simulink model "ofdm_txrx_mimo"  (Simulink 7.1)
settings: Solver=ode45  StartTime=0.0  StopTime=6e3  RelTol=1e-3
PreLoadFcn:
  | ofdm_rx_mimo_init
InitFcn:
  | ofdm_rx_mimo_init
StartFcn:
  | ofdm_rx_mimo_init
values from neighbouring files (symbols this model uses):
  BER_RAM_init_size = 2048    [ofdm_tx_mimo_init.m]
  CPLength = 16    [ofdm_tx_mimo_init.m]
  CRCPolynomial = hex2dec('04c11db7')    [ofdm_tx_mimo_init.m]
  freqOffset_track_filtCoef_i = hex2dec('2500')/2^32    [ofdm_rx_mimo_init.m]
  freqOffset_track_filtCoef_p = hex2dec('d0000')/2^32    [ofdm_rx_mimo_init.m]
  hi = zeros(1,length(cci))    [ofdm_rx_mimo_init.m]
  hr = zeros(1,length(ccr))    [ofdm_rx_mimo_init.m]
  max_CP_length = 16    [ofdm_tx_mimo_init.m]
  max_num_baseRateSymbols = 31    [ofdm_tx_mimo_init.m]
  max_num_subcarriers = 64    [ofdm_tx_mimo_init.m]
  max_num_trainingSymbols = 15    [ofdm_tx_mimo_init.m]
  max_numBytes = 16384    [ofdm_tx_mimo_init.m]
  max_OFDM_symbols = 2047    [ofdm_tx_mimo_init.m]
  modConstellation_qam16 = .75*[1 3 -1 -3]./3    [ofdm_tx_mimo_init.m]
  modConstellation_qam256 = 0.9375*[3 1 5 7 11 9 13 15 -3 -1 -5 -7 -11 -9 -13 -15]./15    [ofdm_tx_mimo_init.m]
  modConstellation_qam64 = 0.875*[3 1 5 7 -3 -1 -5 -7]./7    [ofdm_tx_mimo_init.m]
  modConstellation_qpsk = [1 -1]./sqrt(2)    [ofdm_tx_mimo_init.m]
  numBaseRateSymbols = 2    [ofdm_tx_mimo_init.m]
  numSubcarriers = 64    [ofdm_tx_mimo_init.m]
  numTrainingSymbols = 2    [ofdm_tx_mimo_init.m]
  pilot1_indicies = 7 + ( (64-7) * 2^16)    (= 3735559)    [ofdm_tx_mimo_init.m]
  pilot2_indicies = 21 + ( (64-21) * 2^16)    (= 2818069)    [ofdm_tx_mimo_init.m]
  pilotValue_neg = hex2dec('8000') + (2^16 * 0)    [ofdm_tx_mimo_init.m]
  pilotValue_pos = hex2dec('7FFF') + (2^16 * 0)    [ofdm_tx_mimo_init.m]
  pkt_crossCorr_thresh = 0.7    [ofdm_rx_mimo_init.m]
  pkt_energy_thresh = 0    [ofdm_rx_mimo_init.m]
  pkt_numPayloadBytes = 1400+42-6    (= 1436)    [ofdm_tx_mimo_init.m]
  pktByteNums = numHeaderBytes +  				byteIndex_numPayloadBytes(1)*2^8 +  				byteIndex_numPayloadBytes(2)*2^16 +  				byteIndex_simpleDynModMasks * 2^24    [ofdm_rx_mimo_init.m]
  PktDet_Delay = 26 + Symbol_Timing_Offset*2^7    (= 1050)    [ofdm_rx_mimo_init.m]
  preamble = 6*[0 shortsyms_10 longsyms_2]    [ofdm_tx_mimo_init.m]
  preamble_I = real(preamble)    [ofdm_tx_mimo_init.m]
  preamble_Q = imag(preamble)    [ofdm_tx_mimo_init.m]
  RAM_init_size = max_numBytes    (= 16384)    [ofdm_tx_mimo_init.m]
  reg_Interrupt_PktBuf_Control = reg_InterruptControl +  	1 * 2^16 +  	0 * 2^24    (= 65656)    [ofdm_rx_mimo_init.m]
  rx_controlBits = 1 * 2  		 + 1 * 4  		 + 0 * 8  		 + 1 * 16 * rx_SISO_Mode  		 + 1 * 32  		 + 1 * 64  		 + 0 * 128  		 + 1 * 256  		 + 0 * 512  		 + 1 * 1024  		 + 0 * 2048  		 + 1 * 4096  		 + 1 * 8192  		 + 0 * 16384  		 + 1 * 65536  		 + 1 * 131072  		 + 0 * 262144  		 + 1 * 524288    [ofdm_rx_mimo_init.m]
  Rx_PhaseNoiseTrack_K = 0.6094    [ofdm_rx_mimo_init.m]
  rx_postEq_scaling = round(rxScaling*2^11) * (1 + 2^16)    [ofdm_rx_mimo_init.m]
  RxDataScrambling_Seq = [40 198 78 63 82 173 102 245 48 111 172 115 147 230 216 93 72 65 62 2 205 242 122 90 128 83 105 97 73 10 5 252]    [ofdm_tx_mimo_init.m]
  simOnly_numSamples = length(preamble)+( (numSubcarriers+CPLength)*(numTrainingSymbols + numBaseRateSymbols + numFullRateSymbols/2) )    [ofdm_tx_mimo_init.m]
  train = [train train]    [ofdm_tx_mimo_init.m]
  tx_controlBits = (antB_preambleShift * 2^4) + (2^0 * tx_SISO_Mode) + 4*0 + 8*1    (= 73)    [ofdm_tx_mimo_init.m]
  TxDataScrambling_Seq = [40 198 78 63 82 173 102 245 48 111 172 115 147 230 216 93 72 65 62 2 205 242 122 90 128 83 105 97 73 10 5 252]    [ofdm_tx_mimo_init.m]
  txReg_symbolCounts = (2^16 * numFullRateSymbols) + (2^8 *numBaseRateSymbols ) + numTrainingSymbols    [ofdm_tx_mimo_init.m]
  TxRx_FFTScaling = bin2dec('010111') + (bin2dec('000101') * 2^6)    [ofdm_tx_mimo_init.m]
undefined symbols (not defined in the file or in its folder): AntA, AntB, BaseRate, CRC_table_gen, gcbh, intfilt, pktTiming_count_payloadStart, pktTiming_symbol_length, xlBlockLoadCallback, xlGetSimulinkPeriod
libraries: xbsIndex_r4 x4212, dspmlti4 x4, dspsigops x4, commchan3 x2, simulink x2, commrflib2 x2, dspsrcs4 x1
(part 6 of 43)

== system ofdm_txrx_mimo/OFDM Tx MIMO/OutputMuxes ==
  note: This block adds the preamble onto the signal
      | according to what the preamble generator block sends it.
  note: Cheap output-enable circuit. Output registers are
      | brought out of reset on the first 0->1 transition in the
      | LSB of Pream Index. In other words, the outputs are
      | enabled when the preamble starts, and disabled when
      | the entire packet is finished.
  note: If CP length is 16, we don't need delay here.
      | But if we have CP less than 16, cause RAMS reading address happens later,
      | so does the data. Then data and Sel are pushed further away from preamble.
  Pream Index : Inport  OutDataType=sfix(16)  OutScaling=2^0
  AntA I : Inport  Port=2  OutDataType=sfix(16)  OutScaling=2^0
  AntA Q : Inport  Port=3  OutDataType=sfix(16)  OutScaling=2^0
  AntB I : Inport  Port=4  OutDataType=sfix(16)  OutScaling=2^0
  AntB Q : Inport  Port=5  OutDataType=sfix(16)  OutScaling=2^0
  vin : Inport  Port=6  OutDataType=sfix(16)  OutScaling=2^0
  Pkt Done : Inport  Port=7  OutDataType=sfix(16)  OutScaling=2^0
  Antenna Output Scope : Scope  NumInputPorts=8
      settings: TimeRange=3000  YMin=-1~-1~0~-0.2~-0.4~-1~0~-5  YMax=1~1~1~0.6~0.4~1~400~5
  Constant1 : xbsIndex_r4/Constant  arith_type=Boolean  const=0  n_bits=1  bin_pt=0  explicit_period=off  period=2  equ=P=C  opselect=C  inp2=PCIN>>17  opr=+  inp1=P  carry=CIN  dbl_ovrd=off  block_type=constant
      settings: block_version=VER_STRING_GOES_HERE
  Constant7 : xbsIndex_r4/Constant  arith_type=Boolean  const=1  n_bits=1  bin_pt=0  explicit_period=off  period=2  equ=P=C  opselect=C  inp2=PCIN>>17  opr=+  inp1=P  carry=CIN  dbl_ovrd=off  block_type=constant
      settings: block_version=VER_STRING_GOES_HERE
  Convert : xbsIndex_r4/Convert  arith_type=Unsigned  n_bits=1  bin_pt=0  quantization=Truncate  overflow=Wrap  latency=0  dbl_ovrd=off  pipeline=off  block_type=convert
      settings: xl_area=[0, 0, 0, 0, 0, 0, 0]  block_version=VER_STRING_GOES_HERE
  Delay1 : xbsIndex_r4/Delay  en=off  latency=1  dbl_ovrd=off  reg_retiming=off  block_type=delay
      settings: infoedit=Hardware notes: A delay line is a chain, each link of which is an SRL16 followed by a flip-flop. If register retiming is enabled, the delay line is a chain of flip-flops.  block_version=VER_STRING_GOES_HERE
  Delay2 : xbsIndex_r4/Delay  en=off  latency=1  dbl_ovrd=off  reg_retiming=off  block_type=delay
      settings: infoedit=Hardware notes: A delay line is a chain, each link of which is an SRL16 followed by a flip-flop. If register retiming is enabled, the delay line is a chain of flip-flops.  block_version=VER_STRING_GOES_HERE
  Down Sample1 : xbsIndex_r4/Down Sample  sample_ratio=2  sample_phase=First Value of Frame  en=off  latency=0  dbl_ovrd=off  block_type=dsamp
      settings: block_version=VER_STRING_GOES_HERE
  Inverter : xbsIndex_r4/Inverter  en=off  latency=0  dbl_ovrd=off  block_type=inv
      settings: xl_area=[0, 0, 0, 0, 0, 0, 0]  block_version=VER_STRING_GOES_HERE
  LSB : xbsIndex_r4/Slice  nbits=1  boolean_output=on  mode=Lower Bit Location + Width  bit1=0  base1=MSB of Input  bit0=0  base0=LSB of Input  dbl_ovrd=off  block_type=slice
      settings: infoedit="Extracts a given range of bits from each input sample and presents it at the output.  The output type is ordinarily unsigned with binary point at zero, but can be Boolean when the slice is one bit wide.<P><P>Hardware notes: In hardware this block costs nothing."  block_version=VER_STRING_GOES_HERE
  Logical1 : xbsIndex_r4/Logical  logical_function=OR  inputs=2  en=off  latency=0  precision=Full  arith_type=Unsigned  n_bits=8  bin_pt=2  align_bp=on  dbl_ovrd=off  block_type=logical
      settings: xl_area=[1 0 0 1 0 0 0]  block_version=VER_STRING_GOES_HERE
  Logical3 : xbsIndex_r4/Logical  logical_function=OR  inputs=2  en=off  latency=0  precision=Full  arith_type=Unsigned  n_bits=8  bin_pt=2  align_bp=on  dbl_ovrd=off  block_type=logical
      settings: xl_area=[1 0 0 1 0 0 0]  block_version=VER_STRING_GOES_HERE
  Mux{,1..3} (4 alike) : xbsIndex_r4/Mux  inputs=2  en=off  latency=0  precision=User Defined  arith_type="Signed  (2's comp)"  n_bits=14  bin_pt=13  quantization=Truncate  overflow=Wrap  dbl_ovrd=off  block_type=mux
      settings: xl_area=[7 0 0 14 0 0 0]  block_version=VER_STRING_GOES_HERE
  Payload Running : xbsIndex_r4/Register  init=0  rst=on  en=on  dbl_ovrd=off  block_type=register
      settings: xl_area=[1 1 0 0 0 0 0]  block_version=VER_STRING_GOES_HERE
  Payload Running1 : xbsIndex_r4/Register  init=1  rst=on  en=on  dbl_ovrd=off  block_type=register
      settings: xl_area=[1 1 0 0 0 0 0]  block_version=VER_STRING_GOES_HERE
  Preamble Generation : Subsystem
  Register{,1..3} (4 alike) : xbsIndex_r4/Register  init=0  rst=on  en=off  dbl_ovrd=off  block_type=register
      settings: xl_area=[0, 0, 0, 0, 0, 0, 0]  block_version=VER_STRING_GOES_HERE
  Saturating Scaling : Subsystem
  Tx_debug_pktRunning : xbsIndex_r4/Gateway Out  hdl_port=on  timing_constraint=None  locs_specified=off  LOCs={}  block_type=gatewayout
      settings: infoedit="Gateway out block.  Converts Xilinx fixed point inputs into ouputs of type Simulink integer, double, or fixed point.<P><P>Hardware notes:  In hardware these blocks become top level output ports or are discarded, depending on how they are configured."  xl_area=[0, 0, 0, 0, 0, 0, 0]  sginterface={'Xilinx'=>{'jtaghwcosim'=>{'non_memory_mapped_port'=>'debug_pktRunning'}}}  block_version=VER_STRING_GOES_HERE
  Up Sample : xbsIndex_r4/Up Sample  sample_ratio=4  copy_samples=on  dbl_ovrd=off  block_type=usamp
      settings: infoedit="Up samples input data.  Inserted values  can be zeros or copies of the most recent input sample.<P><P>Hardware notes: No hardware is needed if inserted values are copies of the input sample; otherwise, a mux and single bit flip-flop are used."  block_version=8.2.02
  " AntA I" : Outport  OutDataType=sfix(16)  OutScaling=2^0
  " AntA Q" : Outport  Port=2  OutDataType=sfix(16)  OutScaling=2^0
  " AntB I" : Outport  Port=3  OutDataType=sfix(16)  OutScaling=2^0
  " AntB Q" : Outport  Port=4  OutDataType=sfix(16)  OutScaling=2^0
  tag scope: Tx_Running=global
  wires:
  Logical1/1 -> Mux/1, Mux1/1, Mux2/1, Mux3/1, Antenna Output Scope/6, Delay2/1
  Constant7/1 -> Payload Running/1
  [TxReset] -> Logical3/1
  Logical3/1 -> Down Sample1/1
  Pkt Done/1 -> Logical3/2
  vin/1 -> Payload Running/3, Logical1/2
  AntA I/1 -> Mux/3, Antenna Output Scope/7
  Mux/1 -> Register/1
  Mux1/1 -> Register1/1
  AntA Q/1 -> Mux1/3, Antenna Output Scope/8
  Constant1/1 -> Payload Running1/1
  Payload Running1/1 -> Register/2, Register1/2, Register2/2, Register3/2, Antenna Output Scope/3, Inverter/1
  LSB/1 -> Payload Running1/3
  Pream Index/1 -> LSB/1, Preamble Generation/1(Addr)
  Preamble Generation/1(AntA_I) -> Mux/2, Antenna Output Scope/4
  Preamble Generation/2(AntA_Q) -> Mux1/2, Antenna Output Scope/5
  Register/1 -> Antenna Output Scope/1, Saturating Scaling/2(AntA I)
  Register1/1 -> Antenna Output Scope/2, Saturating Scaling/3(AntA Q)
  Mux2/1 -> Register2/1
  Mux3/1 -> Register3/1
  Register2/1 -> Saturating Scaling/4(AntB I)
  Register3/1 -> Saturating Scaling/5(AntB Q)
  AntB I/1 -> Mux2/3
  AntB Q/1 -> Mux3/3
  Preamble Generation/3(AntB_I) -> Mux2/2
  Preamble Generation/4(AntB_Q) -> Mux3/2
  Down Sample1/1 -> Payload Running/2, Delay1/1
  Payload Running/1 -> Logical1/1
  Delay1/1 -> Payload Running1/2
  Inverter/1 -> Convert/1, [Tx_Running]
  Convert/1 -> Up Sample/1
  Up Sample/1 -> Tx_debug_pktRunning/1
  Saturating Scaling/1(AntA_I) -> " AntA I"/1
  Saturating Scaling/2(AntA_Q) -> " AntA Q"/1
  Saturating Scaling/3(AntB_I) -> " AntB I"/1
  Saturating Scaling/4(AntB_Q) -> " AntB Q"/1
  Delay2/1 -> Saturating Scaling/1(Payload)

== system ofdm_txrx_mimo/OFDM Tx MIMO/Training_Data ==
  CPn_Data : Inport  OutDataType=sfix(16)  OutScaling=2^0
  train_pay : Inport  Port=2  OutDataType=sfix(16)  OutScaling=2^0
  BaseRate_En : Inport  Port=3  OutDataType=sfix(16)  OutScaling=2^0
  FFT En : Inport  Port=4  OutDataType=sfix(16)  OutScaling=2^0
  Start : Inport  Port=5  OutDataType=sfix(16)  OutScaling=2^0
  packet_done : Inport  Port=6  OutDataType=sfix(16)  OutScaling=2^0
  xn_index : Inport  Port=7  OutDataType=sfix(16)  OutScaling=2^0
  Ant Selector : Subsystem
  Convert2 : xbsIndex_r4/Convert  arith_type="Signed  (2's comp)"  n_bits=16  bin_pt=15  quantization=Truncate  overflow=Wrap  latency=0  dbl_ovrd=off  pipeline=off  block_type=convert
      settings: xl_area=[0 0 0 0 0 0 0]  block_version=VER_STRING_GOES_HERE
  Convert3 : xbsIndex_r4/Convert  arith_type="Signed  (2's comp)"  n_bits=16  bin_pt=15  quantization=Truncate  overflow=Wrap  latency=0  dbl_ovrd=off  pipeline=off  block_type=convert
      settings: xl_area=[0 0 0 0 0 0 0]  block_version=VER_STRING_GOES_HERE
  Delay2 : xbsIndex_r4/Delay  en=off  latency=1  dbl_ovrd=off  reg_retiming=off  block_type=delay
      settings: infoedit=Hardware notes: A delay line is a chain, each link of which is an SRL16 followed by a flip-flop. If register retiming is enabled, the delay line is a chain of flip-flops.  xl_area=[1 1 0 0 0 0 0]  block_version=VER_STRING_GOES_HERE
  FlexibleMod : Subsystem
  Logical : xbsIndex_r4/Logical  logical_function=AND  inputs=3  en=off  latency=0  precision=Full  arith_type=Unsigned  n_bits=8  bin_pt=2  align_bp=on  dbl_ovrd=off  block_type=logical
      settings: xl_area=[0, 0, 0, 0, 0, 0, 0]  block_version=VER_STRING_GOES_HERE
  Logical1 : xbsIndex_r4/Logical  logical_function=OR  inputs=2  en=off  latency=0  precision=Full  arith_type=Unsigned  n_bits=8  bin_pt=2  align_bp=on  dbl_ovrd=off  block_type=logical
      settings: xl_area=[0, 0, 0, 0, 0, 0, 0]  block_version=VER_STRING_GOES_HERE
  Logical5 : xbsIndex_r4/Logical  logical_function=AND  inputs=2  en=off  latency=0  precision=Full  arith_type=Unsigned  n_bits=8  bin_pt=2  align_bp=on  dbl_ovrd=off  block_type=logical
      settings: xl_area=[0, 0, 0, 0, 0, 0, 0]  block_version=VER_STRING_GOES_HERE
  Training_Pilots : Subsystem
  tx_Q : Outport  OutDataType=sfix(16)  OutScaling=2^0
  tx_I : Outport  Port=2  OutDataType=sfix(16)  OutScaling=2^0
  wires:
  FlexibleMod/2(Q) -> Training_Pilots/3(Q_mod)
  FlexibleMod/1(I) -> Training_Pilots/2(I_mod)
  packet_done/1 -> FlexibleMod/2(packet_done), Logical1/1
  xn_index/1 -> FlexibleMod/1(ifft_index), Training_Pilots/1(Xn_index)
  train_pay/1 -> Delay2/1
  Logical/1 -> FlexibleMod/3(vin)
  Start/1 -> FlexibleMod/4(start)
  BaseRate_En/1 -> Ant Selector/1(BaseRate), Logical5/2
  CPn_Data/1 -> Ant Selector/2(CP#_Data), Logical/2
  Delay2/1 -> Logical5/1, Training_Pilots/4(train#), Logical/3
  Logical5/1 -> FlexibleMod/5(BaseRate_En)
  Ant Selector/1(Sel) -> FlexibleMod/6(ant_sel), Training_Pilots/5(antSel)
  FFT En/1 -> Logical/1
  Training_Pilots/1(tx_I) -> Convert3/1
  Training_Pilots/2(tx_Q) -> Convert2/1
  Convert3/1 -> tx_I/1
  Convert2/1 -> tx_Q/1
  Logical1/1 -> Training_Pilots/6(rst)
  [TxReset] -> Logical1/2

== system ofdm_txrx_mimo/OFDM Tx MIMO/Tx Reset Logic ==
  Constant3 : Constant  Value=0  OutDataType=sfix(16)  OutScaling=2^0
  Logical : xbsIndex_r4/Logical  logical_function=OR  inputs=2  en=off  latency=0  precision=Full  arith_type=Unsigned  n_bits=16  bin_pt=0  align_bp=on  dbl_ovrd=off  block_type=logical
      settings: block_version=VER_STRING_GOES_HERE
  ToBool : xbsIndex_r4/Convert  arith_type=Boolean  n_bits=16  bin_pt=14  quantization=Truncate  overflow=Wrap  latency=0  dbl_ovrd=off  pipeline=off  block_type=convert
      settings: block_version=VER_STRING_GOES_HERE
  Tx_Reset : xbsIndex_r4/Gateway In  arith_type=Unsigned  n_bits=1  bin_pt=0  quantization="Round  (unbiased: +/- Inf)"  overflow=Saturate  period=4  dbl_ovrd=off  timing_constraint=None  locs_specified=off  LOCs={}  block_type=gatewayin
      settings: infoedit="Gateway in block.  Converts inputs of type Simulink integer, double and fixed point to  Xilinx fixed point type.<P><P>Hardware notes:  In hardware these blocks become top level input ports."  xl_area=[0, 0, 0, 0, 0, 0, 0]  block_version=VER_STRING_GOES_HERE
  tag scope: TxReset=global
  wires:
  Tx_Reset/1 -> Logical/1
  Logical/1 -> ToBool/1
  ToBool/1 -> [TxReset]
  Constant3/1 -> Tx_Reset/1
  [TxReg_TxReset] -> Logical/2

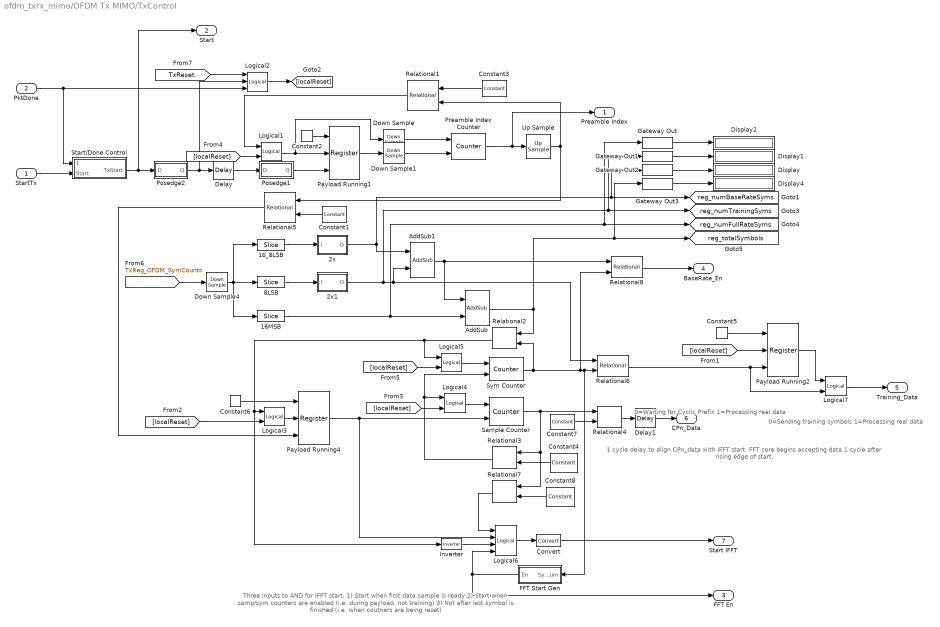
== system ofdm_txrx_mimo/OFDM Tx MIMO/TxControl ==
  note: 0=Waiting for Cyclic Prefix
      | 1=Processing real data
  note: 0=Sending training symbols
      | 1=Processing real data
  note: 1 cycle delay to align CPn_data
      | with IFFT start. FFT core begins
      | accepting data 1 cycle after
      | rising edge of start.
  note: Three inputs to AND for IFFT start:
      | 1) Start when first data sample is ready
      | 2) Start when samp/sym counters are enabled
      | (i.e. during payload, not training)
      | 3) Not after last symbol is finished
      | (i.e. when coutners are being reset)
  StartTx : Inport  OutDataType=sfix(16)  OutScaling=2^0
  PktDone : Inport  Port=2  OutDataType=sfix(16)  OutScaling=2^0
  16MSB : xbsIndex_r4/Slice  nbits=ceil(log2(max_OFDM_symbols))  boolean_output=off  mode=Lower Bit Location + Width  bit1=0  base1=MSB of Input  bit0=16  base0=LSB of Input  dbl_ovrd=off  block_type=slice
      settings: infoedit="Extracts a given range of bits from each input sample and presents it at the output.  The output type is ordinarily unsigned with binary point at zero, but can be Boolean when the slice is one bit wide.<P><P>Hardware notes: In hardware this block costs nothing."  block_version=VER_STRING_GOES_HERE
  16_8LSB : xbsIndex_r4/Slice  nbits=ceil(log2(max_num_baseRateSymbols+1))  boolean_output=off  mode=Lower Bit Location + Width  bit1=0  base1=MSB of Input  bit0=8  base0=LSB of Input  dbl_ovrd=off  block_type=slice
      settings: infoedit="Extracts a given range of bits from each input sample and presents it at the output.  The output type is ordinarily unsigned with binary point at zero, but can be Boolean when the slice is one bit wide.<P><P>Hardware notes: In hardware this block costs nothing."  block_version=VER_STRING_GOES_HERE
  2x : Subsystem
  2x1 : Subsystem
  8LSB : xbsIndex_r4/Slice  nbits=ceil(log2(max_num_trainingSymbols+1))  boolean_output=off  mode=Lower Bit Location + Width  bit1=0  base1=MSB of Input  bit0=0  base0=LSB of Input  dbl_ovrd=off  block_type=slice
      settings: infoedit="Extracts a given range of bits from each input sample and presents it at the output.  The output type is ordinarily unsigned with binary point at zero, but can be Boolean when the slice is one bit wide.<P><P>Hardware notes: In hardware this block costs nothing."  block_version=VER_STRING_GOES_HERE
  AddSub : xbsIndex_r4/AddSub  mode=Addition  use_carryin=off  use_carryout=off  en=off  latency=0  precision=Full  arith_type=Unsigned  n_bits=10  bin_pt=0  quantization=Truncate  overflow=Wrap  dbl_ovrd=off  use_behavioral_HDL=on  pipelined=off  use_rpm=on  hw_selection=Fabric  block_type=addsub
      settings: xl_area=[0, 0, 0, 0, 0, 0, 0]  block_version=VER_STRING_GOES_HERE
  AddSub1 : xbsIndex_r4/AddSub  mode=Addition  use_carryin=off  use_carryout=off  en=off  latency=0  precision=Full  arith_type=Unsigned  n_bits=10  bin_pt=0  quantization=Truncate  overflow=Wrap  dbl_ovrd=off  use_behavioral_HDL=on  pipelined=off  use_rpm=on  hw_selection=Fabric  block_type=addsub
      settings: xl_area=[0, 0, 0, 0, 0, 0, 0]  block_version=VER_STRING_GOES_HERE
  Constant1 : xbsIndex_r4/Constant  Orientation=left  arith_type=Unsigned  const=length(preamble)-158  n_bits=ceil(log2(length(preamble)))  bin_pt=0  explicit_period=off  period=1  equ=P=C  opselect=C  inp2=PCIN>>17  opr=+  inp1=P  carry=CIN  dbl_ovrd=off  block_type=constant
      settings: block_version=VER_STRING_GOES_HERE
  Constant2 : xbsIndex_r4/Constant  arith_type=Boolean  const=1  n_bits=1  bin_pt=0  explicit_period=off  period=1  equ=P=C  opselect=C  inp2=PCIN>>17  opr=+  inp1=P  carry=CIN  dbl_ovrd=off  block_type=constant
      settings: block_version=VER_STRING_GOES_HERE
  Constant3 : xbsIndex_r4/Constant  Orientation=left  arith_type=Unsigned  const=length(preamble)-1  n_bits=ceil(log2(length(preamble)))  bin_pt=0  explicit_period=off  period=1  equ=P=C  opselect=C  inp2=PCIN>>17  opr=+  inp1=P  carry=CIN  dbl_ovrd=off  block_type=constant
      settings: block_version=VER_STRING_GOES_HERE
  Constant4 : xbsIndex_r4/Constant  Orientation=left  arith_type=Unsigned  const=numSubcarriers+CPLength-1  n_bits=ceil(log2(max_num_subcarriers+CPLength+1))  bin_pt=0  explicit_period=off  period=1  equ=P=C  opselect=C  inp2=PCIN>>17  opr=+  inp1=P  carry=CIN  dbl_ovrd=off  block_type=constant
      settings: block_version=VER_STRING_GOES_HERE
  Constant5 : xbsIndex_r4/Constant  arith_type=Boolean  const=1  n_bits=1  bin_pt=0  explicit_period=off  period=1  equ=P=C  opselect=C  inp2=PCIN>>17  opr=+  inp1=P  carry=CIN  dbl_ovrd=off  block_type=constant
      settings: block_version=VER_STRING_GOES_HERE
  Constant6 : xbsIndex_r4/Constant  arith_type=Boolean  const=1  n_bits=1  bin_pt=0  explicit_period=off  period=1  equ=P=C  opselect=C  inp2=PCIN>>17  opr=+  inp1=P  carry=CIN  dbl_ovrd=off  block_type=constant
      settings: AttributesFormatString=%<explicit_period>  block_version=VER_STRING_GOES_HERE
  Constant7 : xbsIndex_r4/Constant  arith_type=Unsigned  const=numSubcarriers-1  n_bits=log2(numSubcarriers)  bin_pt=0  explicit_period=off  period=1  equ=P=C  opselect=C  inp2=PCIN>>17  opr=+  inp1=P  carry=CIN  dbl_ovrd=off  block_type=constant
      settings: block_version=VER_STRING_GOES_HERE
  Constant8 : xbsIndex_r4/Constant  Orientation=left  arith_type=Unsigned  const=0  n_bits=1  bin_pt=0  explicit_period=off  period=1  equ=P=C  opselect=C  inp2=PCIN>>17  opr=+  inp1=P  carry=CIN  dbl_ovrd=off  block_type=constant
      settings: block_version=VER_STRING_GOES_HERE
  Convert : xbsIndex_r4/Convert  arith_type=Boolean  n_bits=8  bin_pt=6  quantization=Truncate  overflow=Wrap  latency=0  dbl_ovrd=off  pipeline=off  block_type=convert
      settings: xl_area=[0, 0, 0, 0, 0, 0, 0]  block_version=VER_STRING_GOES_HERE
  Delay : xbsIndex_r4/Delay  en=off  latency=1  dbl_ovrd=off  reg_retiming=off  block_type=delay
      settings: infoedit=Hardware notes: A delay line is a chain, each link of which is an SRL16 followed by a flip-flop. If register retiming is enabled, the delay line is a chain of flip-flops.  xl_area=[1 1 0 0 0 0 0]  block_version=VER_STRING_GOES_HERE
  Delay1 : xbsIndex_r4/Delay  en=off  latency=1  dbl_ovrd=off  reg_retiming=off  block_type=delay
      settings: infoedit=Hardware notes: A delay line is a chain, each link of which is an SRL16 followed by a flip-flop. If register retiming is enabled, the delay line is a chain of flip-flops.  xl_area=[1 1 0 0 0 0 0]  block_version=VER_STRING_GOES_HERE
  Display{,1..2,4} (4 alike) : Display
  Down Sample : xbsIndex_r4/Down Sample  sample_ratio=2  sample_phase=First Value of Frame  en=off  latency=0  dbl_ovrd=off  block_type=dsamp
      settings: block_version=VER_STRING_GOES_HERE
  Down Sample1 : xbsIndex_r4/Down Sample  sample_ratio=2  sample_phase=First Value of Frame  en=off  latency=0  dbl_ovrd=off  block_type=dsamp
      settings: block_version=VER_STRING_GOES_HERE
  Down Sample4 : xbsIndex_r4/Down Sample  sample_ratio=2  sample_phase="Last Value of Frame  (most efficient)"  en=off  latency=1  dbl_ovrd=off  block_type=dsamp
      settings: block_version=VER_STRING_GOES_HERE
  FFT Start Gen : Subsystem  Orientation=left
  Gateway Out{,1..3} (4 alike) : xbsIndex_r4/Gateway Out  hdl_port=off  timing_constraint=None  locs_specified=off  LOCs={}  block_type=gatewayout
      settings: infoedit="Gateway out block.  Converts Xilinx fixed point inputs into ouputs of type Simulink integer, double, or fixed point.<P><P>Hardware notes:  In hardware these blocks become top level output ports or are discarded, depending on how they are configured."  block_version=VER_STRING_GOES_HERE
  Inverter : xbsIndex_r4/Inverter  en=off  latency=0  dbl_ovrd=off  block_type=inv
      settings: xl_area=[0, 0, 0, 0, 0, 0, 0]  block_version=VER_STRING_GOES_HERE
  Logical{1,3..5,7} (5 alike) : xbsIndex_r4/Logical  logical_function=OR  inputs=2  en=off  latency=0  precision=Full  arith_type=Unsigned  n_bits=8  bin_pt=2  align_bp=on  dbl_ovrd=off  block_type=logical
      settings: xl_area=[1 0 0 1 0 0 0]  block_version=VER_STRING_GOES_HERE
  Logical2 : xbsIndex_r4/Logical  logical_function=OR  inputs=3  en=off  latency=0  precision=Full  arith_type=Unsigned  n_bits=8  bin_pt=2  align_bp=on  dbl_ovrd=off  block_type=logical
      settings: xl_area=[1 0 0 1 0 0 0]  block_version=VER_STRING_GOES_HERE
  Logical6 : xbsIndex_r4/Logical  logical_function=AND  inputs=4  en=off  latency=0  precision=Full  arith_type=Unsigned  n_bits=1  bin_pt=0  align_bp=on  dbl_ovrd=off  block_type=logical
      settings: xl_area=[1 0 0 1 0 0 0]  block_version=VER_STRING_GOES_HERE
  Payload Running1 : xbsIndex_r4/Register  init=0  rst=on  en=on  dbl_ovrd=off  block_type=register
      settings: xl_area=[1 1 0 0 0 0 0]  block_version=VER_STRING_GOES_HERE
  Payload Running2 : xbsIndex_r4/Register  init=0  rst=on  en=on  dbl_ovrd=off  block_type=register
      settings: xl_area=[1 1 0 0 0 0 0]  block_version=VER_STRING_GOES_HERE
  Payload Running4 : xbsIndex_r4/Register  init=0  rst=on  en=on  dbl_ovrd=off  block_type=register
      settings: xl_area=[1 1 0 0 0 0 0]  block_version=VER_STRING_GOES_HERE
  Posedge1 : Subsystem
  Posedge2 : Subsystem
  Preamble Index Counter : xbsIndex_r4/Counter  cnt_type=Free Running  cnt_to=Inf  operation=Up  start_count=0  cnt_by_val=1  arith_type=Unsigned  n_bits=ceil(log2(1+length(preamble_Q)))  bin_pt=0  load_pin=off  rst=on  en=on  explicit_period=off  period=1  dbl_ovrd=off  use_behavioral_HDL=off  use_rpm=on  implementation=Fabric  block_type=counter
      settings: xl_area=[5 9 0 9 0 0 0]  block_version=VER_STRING_GOES_HERE
  Relational{1..3,5,7} (5 alike) : xbsIndex_r4/Relational  Orientation=left  mode=a=b  en=off  latency=0  dbl_ovrd=off  block_type=relational
      Relational1 settings: xl_area=[3 0 0 5 0 0 0]  block_version=VER_STRING_GOES_HERE
      Relational2 settings: xl_area=[2 0 0 4 0 0 0]  block_version=VER_STRING_GOES_HERE
      Relational3 settings: xl_area=[2 0 0 4 0 0 0]  block_version=VER_STRING_GOES_HERE
      Relational5 settings: xl_area=[3 0 0 5 0 0 0]  block_version=VER_STRING_GOES_HERE
      Relational7 settings: xl_area=[2 0 0 4 0 0 0]  block_version=VER_STRING_GOES_HERE
  Relational4 : xbsIndex_r4/Relational  mode=a<=b  en=off  latency=0  dbl_ovrd=off  block_type=relational
      settings: xl_area=[2 0 0 4 0 0 0]  block_version=VER_STRING_GOES_HERE
  Relational6 : xbsIndex_r4/Relational  mode=a<=b  en=off  latency=0  dbl_ovrd=off  block_type=relational
      settings: xl_area=[2 0 0 4 0 0 0]  block_version=VER_STRING_GOES_HERE
  Relational8 : xbsIndex_r4/Relational  mode=a>b  en=off  latency=0  dbl_ovrd=off  block_type=relational
      settings: xl_area=[2 0 0 4 0 0 0]  block_version=VER_STRING_GOES_HERE
  Sample Counter : xbsIndex_r4/Counter  cnt_type=Free Running  cnt_to=Inf  operation=Up  start_count=0  cnt_by_val=1  arith_type=Unsigned  n_bits=1+ceil(log2(numSubcarriers + CPLength + 1))  bin_pt=0  load_pin=off  rst=on  en=on  explicit_period=off  period=1  dbl_ovrd=off  use_behavioral_HDL=off  use_rpm=on  implementation=Fabric  block_type=counter
      settings: xl_area=[4 7 0 7 0 0 0]  block_version=VER_STRING_GOES_HERE
  Start/Done Control : Subsystem
  Sym Counter : xbsIndex_r4/Counter  cnt_type=Free Running  cnt_to=Inf  operation=Up  start_count=0  cnt_by_val=1  arith_type=Unsigned  n_bits=ceil(log2(max_OFDM_symbols+1))  bin_pt=0  load_pin=off  rst=on  en=on  explicit_period=off  period=1  dbl_ovrd=off  use_behavioral_HDL=off  use_rpm=on  implementation=Fabric  block_type=counter
      settings: xl_area=[4 7 0 7 0 0 0]  block_version=VER_STRING_GOES_HERE
  Up Sample : xbsIndex_r4/Up Sample  sample_ratio=2  copy_samples=on  dbl_ovrd=off  block_type=usamp
      settings: infoedit="Up samples input data.  Inserted values  can be zeros or copies of the most recent input sample.<P><P>Hardware notes: No hardware is needed if inserted values are copies of the input sample; otherwise, a mux and single bit flip-flop are used."  block_version=VER_STRING_GOES_HERE
  Preamble Index : Outport  OutDataType=sfix(16)  OutScaling=2^0
  Start : Outport  Port=2  OutDataType=sfix(16)  OutScaling=2^0
  FFT En : Outport  Port=3  OutDataType=sfix(16)  OutScaling=2^0
  BaseRate_En : Outport  Port=4  OutDataType=sfix(16)  OutScaling=2^0
  Training_Data : Outport  Port=5  OutDataType=sfix(16)  OutScaling=2^0
  CPn_Data : Outport  Port=6  OutDataType=sfix(16)  OutScaling=2^0
  Start IFFT : Outport  Port=7  OutDataType=sfix(16)  OutScaling=2^0
  tag scope: reg_numBaseRateSyms=global, reg_numFullRateSyms=global, reg_numTrainingSyms=global, reg_totalSymbols=global
  wires:
  Payload Running1/1 -> Down Sample1/1
  Constant2/1 -> Payload Running1/1
  Logical1/1 -> Payload Running1/2, Down Sample/1
  [localReset] -> Logical1/2
  Preamble Index Counter/1 -> Up Sample/1, Preamble Index/1
  Relational1/1 -> Logical1/1
  Constant3/1 -> Relational1/1
  Posedge1/1(Q) -> Payload Running1/3
  Sample Counter/1 -> Relational4/1, Relational3/1, Relational7/1
  Relational3/1 -> Sym Counter/2, Logical4/1
  Constant6/1 -> Payload Running4/1
  Logical3/1 -> Payload Running4/2
  [localReset] -> Logical3/2
  Payload Running4/1 -> Sample Counter/2, Logical6/2
  Logical4/1 -> Sample Counter/1
  [localReset] -> Logical4/2
  Logical5/1 -> Sym Counter/1
  [localReset] -> Logical5/2
  Sym Counter/1 -> Relational2/2, Relational8/2, Relational6/2, FFT Start Gen/1(SymNum)
  Relational2/1 -> Logical3/1, Inverter/1, Logical5/1
  Relational4/1 -> Delay1/1
  Relational6/1 -> Payload Running2/3, Logical7/2
  Posedge2/1(Q) -> Delay/1, Logical2/2
  Logical2/1 -> [localReset]
  Delay/1 -> Posedge1/1(D)
  Constant1/1 -> Relational5/2
  Relational5/1 -> Payload Running4/3
  [TxReset] -> Logical2/1
  Constant7/1 -> Relational4/2
  PktDone/1 -> Logical2/3, Start/Done Control/1(TxDone)
  Constant5/1 -> Payload Running2/1
  [localReset] -> Payload Running2/2
  Payload Running2/1 -> Logical7/1
  Constant8/1 -> Relational7/2
  Relational7/1 -> Logical6/1
  Logical6/1 -> Convert/1
  Convert/1 -> Start IFFT/1
  Logical7/1 -> Training_Data/1
  Delay1/1 -> CPn_Data/1
  Inverter/1 -> Logical6/3
  Relational8/1 -> BaseRate_En/1
  AddSub1/1 -> Relational8/1, AddSub/1
  Constant4/1 -> Relational3/2
  FFT Start Gen/1(En) -> Logical6/4, FFT En/1
  2x1/1(O) -> [reg_numTrainingSyms], Gateway Out1/1, Relational6/1, AddSub1/2
  2x/1(O) -> AddSub1/1, [reg_numBaseRateSyms], Gateway Out2/1
  AddSub/1 -> Relational2/1, [reg_totalSymbols], Gateway Out3/1
  Gateway Out/1 -> Display2/1
  Gateway Out1/1 -> Display1/1
  Gateway Out2/1 -> Display/1
  Gateway Out3/1 -> Display4/1
  Down Sample/1 -> Preamble Index Counter/1
  Down Sample1/1 -> Preamble Index Counter/2
  Up Sample/1 -> Relational5/1, Relational1/2
  16_8LSB/1 -> 2x/1(I)
  8LSB/1 -> 2x1/1(I)
  16MSB/1 -> AddSub/2, [reg_numFullRateSyms], Gateway Out/1
  [TxReg_OFDM_SymCounts] -> Down Sample4/1
  Down Sample4/1 -> 8LSB/1, 16_8LSB/1, 16MSB/1
  Start/Done Control/1(TxStart) -> Posedge2/1(D), Start/1
  StartTx/1 -> Start/Done Control/2(Start)

== system ofdm_txrx_mimo/OFDM Tx MIMO/TxStart ==
  Copy_Downsample : Subsystem
  Logical : xbsIndex_r4/Logical  logical_function=OR  inputs=2  en=off  latency=0  precision=Full  arith_type=Unsigned  n_bits=16  bin_pt=0  align_bp=on  dbl_ovrd=off  block_type=logical
      settings: block_version=VER_STRING_GOES_HERE
  Pulse Generator1 : DiscretePulseGenerator  Period=(simOnly_numSamples*2 + 500)*2  PhaseDelay=5
  Tx_StartTransmit : xbsIndex_r4/Gateway In  arith_type=Boolean  n_bits=1  bin_pt=0  quantization="Round  (unbiased: +/- Inf)"  overflow=Saturate  period=1  dbl_ovrd=off  timing_constraint=None  locs_specified=off  LOCs={}  block_type=gatewayin
      settings: infoedit="Gateway in block.  Converts inputs of type Simulink integer, double and fixed point to  Xilinx fixed point type.<P><P>Hardware notes:  In hardware these blocks become top level input ports."  xl_area=[0, 0, 0, 0, 0, 0, 0]  block_version=VER_STRING_GOES_HERE
  Start : Outport  OutDataType=sfix(16)  OutScaling=2^0
  wires:
  Tx_StartTransmit/1 -> Copy_Downsample/1(D)
  Pulse Generator1/1 -> Tx_StartTransmit/1
  Logical/1 -> Start/1
  [TxReg_TxStart] -> Logical/2
  Copy_Downsample/1(Q) -> Logical/1
